simulink model "modelo_naolinear"  (R2023a)
settings: Solver=ode4  StartTime=0.0  StopTime=T_sim  FixedStep=dt  RelTol=1e-3  EnableMultiTasking=on
InitFcn:
  | modelo_naolinear_params
values from neighbouring files (symbols this model uses):
  B22 = B(7:12,2:4)    [modelo_naolinear_params.m]
  dt = 1e-3    (= 0.001)    [modelo_naolinear_params.m]
  G = inv(K*B22*inv(R)*B22'-A22')*C'*Q    [modelo_naolinear_params.m]
  g = 9.8    [modelo_naolinear_params.m]
  incluir_din_motor = 1    [modelo_naolinear_params.m]
  input_selector = 1    [modelo_naolinear_params.m]
  Ir = 1e-3    (= 0.001)    [modelo_naolinear_params.m]
  Ixx = 1e-2    (= 0.01)    [modelo_naolinear_params.m]
  Iyy = 1e-2    (= 0.01)    [modelo_naolinear_params.m]
  Izz = 1.8e-2    (= 0.018)    [modelo_naolinear_params.m]
  K = are(A22, B22*inv(R)*B22',C'*Q*C)    [modelo_naolinear_params.m]
  Kd_rot = 0.01    [modelo_naolinear_params.m]
  Kd_trans = -0.13723    [modelo_naolinear_params.m]
  Ki_rot = 1.0    (= 1)    [modelo_naolinear_params.m]
  Ki_trans = 0.4    [modelo_naolinear_params.m]
  Kp = 0.9182    [modelo_naolinear_params.m]
  Kp_rot = 10    [modelo_naolinear_params.m]
  Kp_trans = 2.0968    [modelo_naolinear_params.m]
  m = 1.0428    [modelo_naolinear_params.m]
  N_rot = 100    [modelo_naolinear_params.m]
  N_trans = 5.5815    [modelo_naolinear_params.m]
  p0 = 0    [modelo_naolinear_params.m]
  phi0 = 0    [modelo_naolinear_params.m]
  psi0 = 0    [modelo_naolinear_params.m]
  q0 = 0    [modelo_naolinear_params.m]
  R = diag([r11,r22,r33])    [modelo_naolinear_params.m]
  r0 = 0    [modelo_naolinear_params.m]
  seleciona_controlador = -1    [modelo_naolinear_params.m]
  T = [kf,kf,kf,kf ... (first row only)    [modelo_naolinear_params.m]
  T_inv = T^(-1)    [modelo_naolinear_params.m]
  tau_a = 0.0569    [modelo_naolinear_params.m]
  tau_s = 0.045    [modelo_naolinear_params.m]
  theta0 = 0    [modelo_naolinear_params.m]
  vx0 = 0    [modelo_naolinear_params.m]
  vy0 = 0    [modelo_naolinear_params.m]
  vz0 = 0    [modelo_naolinear_params.m]
  x0 = 0    [modelo_naolinear_params.m]
  y0 = 0    [modelo_naolinear_params.m]
  z0 = 1    [modelo_naolinear_params.m]
undefined symbols (not defined in the file or in its folder): sigbuilder_block
libraries: slpidlib x6, aerolibanimutils x2, sim3dlib x1, uavsim3dlib x1
(part 2 of 2)

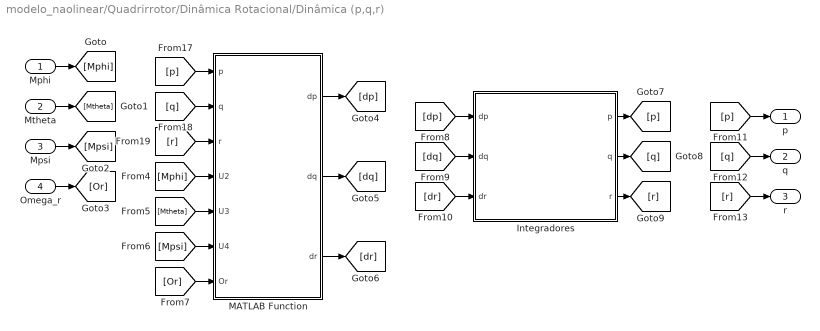
== system modelo_naolinear/Quadrirrotor/Dinâmica Rotacional/Dinâmica (p,q,r) ==
  Mphi : Inport
  Mtheta : Inport  Port=2
  Mpsi : Inport  Port=3
  Omega_r : Inport  Port=4
  Integradores : Subsystem
  MATLAB Function : MATLAB Function  TreatAsAtomicUnit=on
  p : Outport  VectorParamsAs1DForOutWhenUnconnected=off
  q : Outport  Port=2  VectorParamsAs1DForOutWhenUnconnected=off
  r : Outport  Port=3  VectorParamsAs1DForOutWhenUnconnected=off
  wires:
  Mphi/1 -> [Mphi]
  Mtheta/1 -> [Mtheta]
  Mpsi/1 -> [Mpsi]
  Omega_r/1 -> [Or]
  [Or] -> MATLAB Function/7(Or)
  [Mpsi] -> MATLAB Function/6(U4)
  [Mtheta] -> MATLAB Function/5(U3)
  [Mphi] -> MATLAB Function/4(U2)
  MATLAB Function/1(dp) -> [dp]
  MATLAB Function/2(dq) -> [dq]
  [p] -> p/1
  [q] -> q/1
  [r] -> r/1
  [p] -> MATLAB Function/1(p)
  [q] -> MATLAB Function/2(q)
  [r] -> MATLAB Function/3(r)
  MATLAB Function/3(dr) -> [dr]
  [dp] -> Integradores/1(dp)
  [dq] -> Integradores/2(dq)
  [dr] -> Integradores/3(dr)
  Integradores/1(p) -> [p]
  Integradores/2(q) -> [q]
  Integradores/3(r) -> [r]

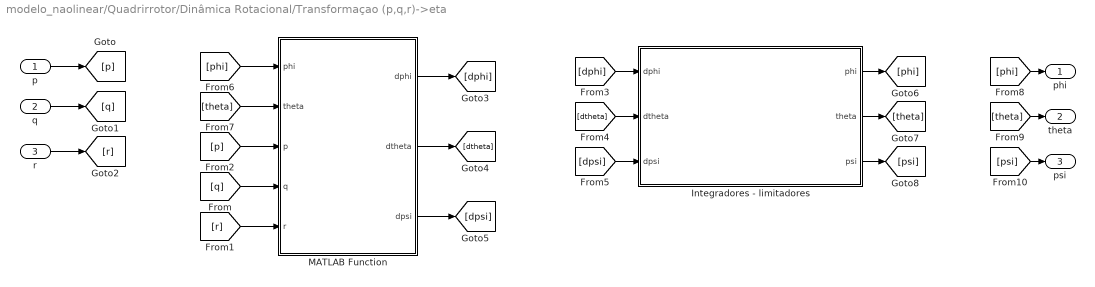
== system modelo_naolinear/Quadrirrotor/Dinâmica Rotacional/Transformaçao (p,q,r)->eta ==
  p : Inport
  q : Inport  Port=2
  r : Inport  Port=3
  Integradores - limitadores : Subsystem
  MATLAB Function : MATLAB Function  TreatAsAtomicUnit=on
  phi : Outport  VectorParamsAs1DForOutWhenUnconnected=off
  theta : Outport  Port=2  VectorParamsAs1DForOutWhenUnconnected=off
  psi : Outport  Port=3  VectorParamsAs1DForOutWhenUnconnected=off
  wires:
  p/1 -> [p]
  q/1 -> [q]
  r/1 -> [r]
  [q] -> MATLAB Function/4(q)
  [r] -> MATLAB Function/5(r)
  [p] -> MATLAB Function/3(p)
  MATLAB Function/1(dphi) -> [dphi]
  MATLAB Function/2(dtheta) -> [dtheta]
  MATLAB Function/3(dpsi) -> [dpsi]
  [phi] -> MATLAB Function/1(phi)
  [theta] -> MATLAB Function/2(theta)
  [phi] -> phi/1
  [theta] -> theta/1
  [psi] -> psi/1
  [dphi] -> Integradores - limitadores/1(dphi)
  [dtheta] -> Integradores - limitadores/2(dtheta)
  [dpsi] -> Integradores - limitadores/3(dpsi)
  Integradores - limitadores/1(phi) -> [phi]
  Integradores - limitadores/2(theta) -> [theta]
  Integradores - limitadores/3(psi) -> [psi]

== system modelo_naolinear/Quadrirrotor/Modelo dos rotores + helices /Motores Brushless DC + Helices  ==
  note: A dinamida pode ser simulada
      | com ou sem modelo dos motores.
  w1d : Inport
  w2d : Inport  Port=2
  w3d : Inport  Port=3
  w4d : Inport  Port=4
  Motor DC 1 : Subsystem
      mask Display:
      | disp('Motor DC')
      | port_label('input',1,'wd')
      | port_label('output',1,'w')
  Motor DC 2 : Subsystem
      mask Display:
      | disp('Motor DC')
      | port_label('input',1,'wd')
      | port_label('output',1,'w')
  Motor DC 3 : Subsystem
      mask Display:
      | disp('Motor DC')
      | port_label('input',1,'wd')
      | port_label('output',1,'w')
  Motor DC 4 : Subsystem
      mask Display:
      | disp('Motor DC')
      | port_label('input',1,'wd')
      | port_label('output',1,'w')
  w1 : Outport  VectorParamsAs1DForOutWhenUnconnected=off
  w2 : Outport  Port=2  VectorParamsAs1DForOutWhenUnconnected=off
  w3 : Outport  Port=3  VectorParamsAs1DForOutWhenUnconnected=off
  w4 : Outport  Port=4  VectorParamsAs1DForOutWhenUnconnected=off
  wires:
  w1d/1 -> Motor DC 1/1(wd)  "w1d"
  w2d/1 -> Motor DC 2/1(In1)  "w2d"
  w3d/1 -> Motor DC 3/1(In1)  "w3d"
  w4d/1 -> Motor DC 4/1(In1)  "w4d"
  Motor DC 1/1(w) -> w1/1  "w1"
  Motor DC 2/1(Out1) -> w2/1  "w2"
  Motor DC 3/1(Out1) -> w3/1  "w3"
  Motor DC 4/1(Out1) -> w4/1  "w4"

== system modelo_naolinear/Quadrirrotor/Modelo dos rotores + helices /Transformaçao U -> w ==
  U1d : Inport
  U2d : Inport  Port=2
  U3d : Inport  Port=3
  U4d : Inport  Port=4
  Bus Creator1 : BusCreator  Inputs=4
  Demux1 : Demux
  Sqrt : Sqrt
  Transformação Omega = T^(-1)*U : Gain  Gain=T_inv  Multiplication=Matrix(K*u)  RndMeth=Nearest  LockScale=on
  w1d : Outport  VectorParamsAs1DForOutWhenUnconnected=off
  w2d : Outport  Port=2  VectorParamsAs1DForOutWhenUnconnected=off
  wd3 : Outport  Port=3  VectorParamsAs1DForOutWhenUnconnected=off
  w4d : Outport  Port=4  VectorParamsAs1DForOutWhenUnconnected=off
  wires:
  Bus Creator1/1 -> Transformação Omega = T^(-1)*U/1
  Transformação Omega = T^(-1)*U/1 -> Sqrt/1
  U3d/1 -> Bus Creator1/3
  U2d/1 -> Bus Creator1/2
  U1d/1 -> Bus Creator1/1
  Demux1/2 -> w2d/1  "w2d"
  U4d/1 -> Bus Creator1/4
  Demux1/3 -> wd3/1  "wd3"
  Demux1/4 -> w4d/1  "w4d"
  Demux1/1 -> w1d/1  "w1d"
  Sqrt/1 -> Demux1/1  "Frequências angulares desejadas"

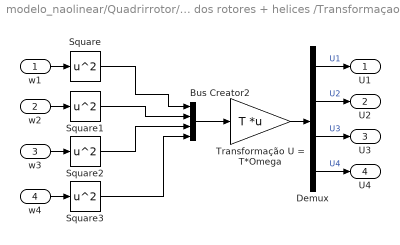
== system modelo_naolinear/Quadrirrotor/Modelo dos rotores + helices /Transformaçao w -> U ==
  w1 : Inport
  w2 : Inport  Port=2
  w3 : Inport  Port=3
  w4 : Inport  Port=4
  Bus Creator2 : BusCreator  Inputs=4
  Demux : Demux
  Square{,1..3} (4 alike) : Math  Operator=square  SignedPower=on
  Transformação U = T*Omega : Gain  Gain=T  Multiplication=Matrix(K*u)  RndMeth=Nearest  LockScale=on
  U1 : Outport  VectorParamsAs1DForOutWhenUnconnected=off
  U2 : Outport  Port=2  VectorParamsAs1DForOutWhenUnconnected=off
  U3 : Outport  Port=3  VectorParamsAs1DForOutWhenUnconnected=off
  U4 : Outport  Port=4  VectorParamsAs1DForOutWhenUnconnected=off
  wires:
  Bus Creator2/1 -> Transformação U = T*Omega/1
  Transformação U = T*Omega/1 -> Demux/1
  Demux/3 -> U3/1  "U3"
  w4/1 -> Square3/1
  w1/1 -> Square/1
  Demux/2 -> U2/1  "U2"
  w2/1 -> Square1/1
  w3/1 -> Square2/1
  Demux/1 -> U1/1  "U1"
  Demux/4 -> U4/1  "U4"
  Square/1 -> Bus Creator2/1
  Square1/1 -> Bus Creator2/2
  Square2/1 -> Bus Creator2/3
  Square3/1 -> Bus Creator2/4

== system modelo_naolinear/Quadrirrotor/Dinâmica Rotacional/Dinâmica (p,q,r)/Integradores ==
  dp : Inport
  dq : Inport  Port=2
  dr : Inport  Port=3
  Integrator3 : Integrator  InitialCondition=p0
  Integrator4 : Integrator  InitialCondition=q0
  Integrator5 : Integrator  InitialCondition=r0
  p : Outport  VectorParamsAs1DForOutWhenUnconnected=off
  q : Outport  Port=2  VectorParamsAs1DForOutWhenUnconnected=off
  r : Outport  Port=3  VectorParamsAs1DForOutWhenUnconnected=off
  wires:
  Integrator3/1 -> p/1
  Integrator4/1 -> q/1
  dp/1 -> Integrator3/1
  dr/1 -> Integrator5/1
  dq/1 -> Integrator4/1
  Integrator5/1 -> r/1

== system modelo_naolinear/Quadrirrotor/Dinâmica Rotacional/Transformaçao (p,q,r)->eta/Integradores - limitadores ==
  dphi : Inport
  dtheta : Inport  Port=2
  dpsi : Inport  Port=3
  -pi<=u<=pi : Fcn  Expr=u - 2*pi*floor( (u+pi)/(2*pi) )
  -pi<=u<=pi1 : Fcn  Expr=u - 2*pi*floor( (u+pi)/(2*pi) )
  -pi<=u<=pi2 : Fcn  Expr=u - 2*pi*floor( (u+pi)/(2*pi) )
  Integrator : Integrator  InitialCondition=phi0
  Integrator1 : Integrator  InitialCondition=theta0
  Integrator2 : Integrator  InitialCondition=psi0
  phi : Outport  VectorParamsAs1DForOutWhenUnconnected=off
  theta : Outport  Port=2  VectorParamsAs1DForOutWhenUnconnected=off
  psi : Outport  Port=3  VectorParamsAs1DForOutWhenUnconnected=off
  wires:
  Integrator/1 -> -pi<=u<=pi/1
  dpsi/1 -> Integrator2/1
  -pi<=u<=pi/1 -> phi/1
  Integrator1/1 -> -pi<=u<=pi1/1
  Integrator2/1 -> -pi<=u<=pi2/1
  -pi<=u<=pi2/1 -> psi/1
  -pi<=u<=pi1/1 -> theta/1
  dphi/1 -> Integrator/1
  dtheta/1 -> Integrator1/1

== system modelo_naolinear/Quadrirrotor/Modelo dos rotores + helices /Motores Brushless DC + Helices /Motor DC 1 ==
  wd : Inport
  Modelo Motor 1 : TransferFcn  Numerator=[Kp]  Denominator=[tau_a 1]
  Transport Delay : TransportDelay  DelayTime=tau_s  PadeOrder=2
  w : Outport  VectorParamsAs1DForOutWhenUnconnected=off
  wires:
  Modelo Motor 1/1 -> w/1  "w1"
  Transport Delay/1 -> Modelo Motor 1/1
  wd/1 -> Transport Delay/1

== system modelo_naolinear/Quadrirrotor/Modelo dos rotores + helices /Motores Brushless DC + Helices /Motor DC 2 ==
  In1 : Inport
  Modelo Motor 2 : TransferFcn  Numerator=[Kp]  Denominator=[tau_a 1]
  Transport Delay : TransportDelay  DelayTime=tau_s  PadeOrder=2
  Out1 : Outport  VectorParamsAs1DForOutWhenUnconnected=off
  wires:
  Transport Delay/1 -> Modelo Motor 2/1
  In1/1 -> Transport Delay/1
  Modelo Motor 2/1 -> Out1/1  "w2"

== system modelo_naolinear/Quadrirrotor/Modelo dos rotores + helices /Motores Brushless DC + Helices /Motor DC 3 ==
  In1 : Inport
  Modelo Motor 3 : TransferFcn  Numerator=[Kp]  Denominator=[tau_a 1]
  Transport Delay : TransportDelay  DelayTime=tau_s  PadeOrder=2
  Out1 : Outport  VectorParamsAs1DForOutWhenUnconnected=off
  wires:
  Modelo Motor 3/1 -> Out1/1  "w3"
  Transport Delay/1 -> Modelo Motor 3/1
  In1/1 -> Transport Delay/1

== system modelo_naolinear/Quadrirrotor/Modelo dos rotores + helices /Motores Brushless DC + Helices /Motor DC 4 ==
  In1 : Inport
  Modelo Motor 4 : TransferFcn  Numerator=[Kp]  Denominator=[tau_a 1]
  Transport Delay : TransportDelay  DelayTime=tau_s  PadeOrder=2
  Out1 : Outport  VectorParamsAs1DForOutWhenUnconnected=off
  wires:
  Transport Delay/1 -> Modelo Motor 4/1
  In1/1 -> Transport Delay/1
  Modelo Motor 4/1 -> Out1/1  "w4"

== matlab function modelo_naolinear/Quadrirrotor/Dinâmica Rotacional/Transformaçao (p,q,r)->eta/MATLAB Function ==
  data: phi input; dphi output; theta input; p input; q input; r input; dtheta output; dpsi output
function [dphi, dtheta, dpsi] = pqr_eta_rhs(phi, theta,p,q,r)

dphi = (r*cos(phi) + q*sin(phi))*tan(theta) + p;
dtheta = q*cos(phi) - r*sin(phi);
dpsi = (cos(phi)/cos(theta))*r + (sin(phi)/cos(theta))*q;
end

== matlab function modelo_naolinear/Quadrirrotor/Dinâmica Rotacional/Dinâmica (p,q,r)/MATLAB Function ==
  data: p input; q input; dp output size=1; r input; U2 input; U3 input; U4 input; Or input; dq output size=1; dr output size=1; Ixx parameter; Iyy parameter; Izz parameter; Ir parameter
function [dp, dq, dr] = pqr_rhs(p,q,r,U2,U3,U4,Or, Ixx, Iyy, Izz, Ir)

dp = (Iyy-Izz)*q*r/Ixx - (Ir*Or)*q/Ixx + U2/Ixx;
dq = (Izz-Ixx)*p*r/Iyy - (Ir*Or)*p/Iyy + U3/Iyy;
dr = (Ixx-Iyy)*p*q/Izz + U4/Izz;
end

== matlab function modelo_naolinear/Quadrirrotor/Dinamica Translacional/MATLAB Function ==
  data: phi input; ddotx output; theta input; psi input; U1 input; ddoty output; ddotz output; m parameter; g parameter
function [ddotx, ddoty, ddotz] = din_trans_rhs(phi, theta, psi, U1, m, g)

ddotx = (cos(phi)*cos(psi)*sin(theta) + sin(phi)*sin(psi))*U1/m;
ddoty = (cos(phi)*sin(psi)*sin(theta) - sin(phi)*cos(psi))*U1/m;
ddotz = -g + cos(phi)*cos(theta)*U1/m;
end

== matlab function modelo_naolinear/Controle Translacional/MATLAB Function ==
  data: u1 input; phi_d output; u2 input; u3 input; theta_d output; psi_d output; U1 output; g parameter; m parameter
function [phi_d, theta_d, psi_d, U1] = entradas_virtuais(u1, u2, u3, g, m)
phi_d = atan(-u2/sqrt(u1^2+(g+u3)^2));
theta_d = atan(u1/(g+u3));
psi_d = 0;
U1 = m*sqrt(u1^2 + u2^2 + (g+u3)^2);
end

== matlab function modelo_naolinear/Controle Rotacional/MATLAB Function ==
  data: p input; Mphi output; q input; r input; phi input; theta input; psi input; phi_d input; theta_d input; psi_d input; Mtheta output; Mpsi output; K parameter; G parameter; R parameter; B22 parameter
function [Mphi, Mtheta, Mpsi] = controlador_lqt(p,q,r,...
    phi,theta,psi,phi_d,theta_d,psi_d, K, G, R, B22)
% controlador Lineqr Quadratic Tranking para a dinâmica rotacional.
% assume-se uma dinâmica linear do tipo: 
%
%                    dx/dt = Ax + Bu
%                        y = Dx
% onde:
%   A é uma matriz 6x6
%   B é uma matriz 6x3
%   C é uma matriz 3x6
%
% note que na saída são observados apenas os ângulos de Euler.
%
% O índice de desempenho é:
%
%       J = (1/2)e'He + (1/2)int_{t_0}^{t_f}(e'Qe + u'Ru)dt
%
% onde:
%   e(t) = z(t) - Cx(t), é o erro a ser minimizado
%   z(t) é a referência a ser seguida.
%   As matrizes H, Q e R são de dimensões apropriadas
%
% O controle ótimo é:
%                
%                      u = R^(-1)*B'*(g - Kx)
% onde:
%   g(t) é a solução algébrica da equação:
%
%                0 = (KBR^(-1)B'-A')g - C'Qz
% ou g = Gz, onde
%                G = (KBR^(-1)B'-A')^(-1)C'Q
%
% A matriz K é a solução da ARE
%
%               A'*K + K*A - K*S*K + V = 0
% onde:
%   S = BR^(-1)B'
%   V = C'QC
% -------------------------------------------------------------------------
x = [p;q;r;phi;theta;psi];
z = [phi_d;theta_d;psi_d];
%
g = G*z;
u = inv(R)*B22'*(g-K*x);
Mphi = u(1);
Mtheta = u(2);
Mpsi = u(3);
end
